simulink model "ofdm_txrx_supermimo"  (Simulink 7.1)
settings: Solver=VariableStepDiscrete  SolverMode=SingleTasking  StartTime=0.0  StopTime=simOnly_simLength  RelTol=1e-3
PostLoadFcn:
  | ofdm_rx_supermimo_init
InitFcn:
  | ofdm_rx_supermimo_init
StartFcn:
  | ofdm_rx_supermimo_init
values from neighbouring files (symbols this model uses):
  Action0_Config = 0* 31*2^18 + 0*2^24 + PHY_AUTORESPONSE_REQ_GOODHDR + PHY_AUTORESPONSE_REQ_GOODPKT + PHY_AUTORESPONSE_REQ_FLAGA    [ofdm_tx_supermimo_init.m]
  Action1_Config = 0* 62*2^18 + PHY_AUTORESPONSE_REQ_GOODHDR + PHY_AUTORESPONSE_REQ_GOODPKT    [ofdm_tx_supermimo_init.m]
  Action2_Config = 0    [ofdm_tx_supermimo_init.m]
  Action3_Config = 0    [ofdm_tx_supermimo_init.m]
  Action4_Config = 0    [ofdm_tx_supermimo_init.m]
  Action5_Config = 0    [ofdm_tx_supermimo_init.m]
  ActionID_AFTransmit = 31    [ofdm_tx_supermimo_init.m]
  ActionID_Disabled = 0    [ofdm_tx_supermimo_init.m]
  ActionID_SetFlagA = 62    [ofdm_tx_supermimo_init.m]
  ActionID_SetFlagB = 61    [ofdm_tx_supermimo_init.m]
  BER_RAM_init_size = 2048    [ofdm_tx_supermimo_init.m]
  CPLength = 16    [ofdm_tx_supermimo_init.m]
  longCorr_coef_i = [-4*real(LTS) 0]    [ofdm_rx_supermimo_init.m]
  longCorr_coef_q = [4*imag(LTS) 0]    [ofdm_rx_supermimo_init.m]
  Match0_Config = 0    [ofdm_tx_supermimo_init.m]
  Match1_Config = 0    [ofdm_tx_supermimo_init.m]
  Match2_Config = 0    [ofdm_tx_supermimo_init.m]
  Match3_Config = 0    [ofdm_tx_supermimo_init.m]
  Match4_Config = 0    [ofdm_tx_supermimo_init.m]
  Match5_Config = 0    [ofdm_tx_supermimo_init.m]
  Match6_Config = 0    [ofdm_tx_supermimo_init.m]
  Match7_Config = 0    [ofdm_tx_supermimo_init.m]
  max_CP_length = 16    [ofdm_tx_supermimo_init.m]
  max_num_baseRateSymbols = 31    [ofdm_tx_supermimo_init.m]
  max_num_subcarriers = 64    [ofdm_tx_supermimo_init.m]
  max_num_trainingSymbols = 15    [ofdm_tx_supermimo_init.m]
  max_numBytes = 16384    [ofdm_tx_supermimo_init.m]
  max_OFDM_symbols = 511    [ofdm_tx_supermimo_init.m]
  modConstellation_qam16 = .75*[1 3 -1 -3]./3    [ofdm_tx_supermimo_init.m]
  modConstellation_qam64 = 0.875*[3 1 5 7 -3 -1 -5 -7]./7    [ofdm_tx_supermimo_init.m]
  modConstellation_qpsk = [1 -1]./sqrt(2)    [ofdm_tx_supermimo_init.m]
  numBaseRateSymbols = ceil(numHeaderBytes / numBytes_BaseRateOFDMSymbol)    [ofdm_tx_supermimo_init.m]
  numSubcarriers = 64    [ofdm_tx_supermimo_init.m]
  numTrainingSymbols = 2    [ofdm_tx_supermimo_init.m]
  pilot_indicies = 7 + ( (64-21) * 2^8) + ( (64-7) * 2^16) + (21 * 2^24)    (= 356068103)    [ofdm_tx_supermimo_init.m]
  pilotValues = hex2dec('A57D') + (2^16 * hex2dec('5A82'))    [ofdm_tx_supermimo_init.m]
  pkt_crossCorr_thresh = 0.7    [ofdm_rx_supermimo_init.m]
  pkt_energy_thresh = 0    [ofdm_rx_supermimo_init.m]
  pktByteNums = numHeaderBytes +  				byteIndex_numPayloadBytes(1)*2^8 +  				byteIndex_numPayloadBytes(2)*2^16 +  				byteIndex_simpleDynModMasks * 2^24    [ofdm_rx_supermimo_init.m]
  PktDet_Delay = 58+32 +      Symbol_Timing_Offset*2^7 +      0*2^16 +      (117* 2^24)    (= 1962935642)    [ofdm_rx_supermimo_init.m]
  pktTiming_count_payloadStart = pktTiming_count_lsStart + pktTiming_ls_num*pktTiming_ls_length    [ofdm_rx_supermimo_init.m]
  preamble = preamble_scale*[shortsyms_10 longsyms_2]    [ofdm_tx_supermimo_init.m]
  preamble_ROM = preamble_I_ROM + preamble_Q_ROM*2^16    [ofdm_tx_supermimo_init.m]
  RAM_init_size = max_numBytes    (= 16384)    [ofdm_tx_supermimo_init.m]
  reg_AF_Tx_Blanking = (320+80) + ( (320+80+79)*2^16)    (= 31392144)    [ofdm_rx_supermimo_init.m]
  reg_AF_Tx_Scaling = 2944    [ofdm_rx_supermimo_init.m]
  reg_Interrupt_PktBuf_Control = reg_InterruptControl +  	1 * 2^16 +  	0 * 2^24    (= 65656)    [ofdm_rx_supermimo_init.m]
  reg_PilotCalcParams = (4 * 2^16) + (minPilotChanMag)    (= 262184.96)    [ofdm_rx_supermimo_init.m]
  regRx_chanEst_minMag = ((0.5)*2^15) + ((0.5 * 2^15)*2^16)    (= 1073758208)    [ofdm_rx_supermimo_init.m]
  regRx_coarseCalcCorrection = round((1.3e-3 - 1.2982e-3)*2^32)    [ofdm_rx_supermimo_init.m]
  regRx_pilotCalcCorrection = round(1.00115 * 2^31)    [ofdm_rx_supermimo_init.m]
  regRx_preCFOoptions = 0 * 2^0 +  	1 * 2^1 +  	0    (= 2)    [ofdm_rx_supermimo_init.m]
  rx_controlBits = 1 * 2  		 + 1 * 4  		 + 0 * 8  		 + 1 * 16 * rx_SISO_Mode  		 + 1 * 32  		 + 1 * 64  		 + 1 * 128  		 + 1 * 256  		 + 1 * 512  		 + 1 * 1024  		 + 1 * 4096  		 + 0 * 16384  		 + 0 * 32768  		 + 1 * 65536  		 + 0 * 131072  		 + 0 * 262144  		 + 1 * 524288  		 + 1 * 1048576 * tx_Alamouti_Mode  		 + 0 * 2097152           + 1 * 33554432  		 + 0 * 4194304  		 + 0 * 8388608  		 + 0 * 16777216  		 + 1 *  ...(+49 chars)    [ofdm_rx_supermimo_init.m]
  Rx_PktDet_LongCorr_Params = LongCorrCounterLoad + 0*2^8 + 0*2^16 + 255*2^24    (= 4278190325)    [ofdm_rx_supermimo_init.m]
  Rx_PktDet_LongCorrThresholds = 8000    [ofdm_rx_supermimo_init.m]
  rx_postEq_scaling = round(rxScaling*2^11) * (1 + 2^16)    [ofdm_rx_supermimo_init.m]
  RxDataScrambling_Seq = [40 198 78 63 82 173 102 245 48 111 172 115 147 230 216 93 72 65 62 2 205 242 122 90 128 83 105 97 73 10 5 252]    [ofdm_tx_supermimo_init.m]
  RxReg_FixedPktLen = 0    [ofdm_rx_supermimo_init.m]
  shortSymbol_time = shortSymbol_time(1:16).'    [ofdm_tx_supermimo_init.m]
  simOnly_numSamples = length(preamble)+( (numSubcarriers+CPLength)*(numTrainingSymbols + numBaseRateSymbols + numFullRateSymbols/2) )    [ofdm_tx_supermimo_init.m]
  train = [train train]    [ofdm_tx_supermimo_init.m]
  tx_controlBits = (antB_preambleShift * 2^4) + (2^1 * tx_Alamouti_Mode) +  	(2^0 * tx_SISO_Mode) + 4*0 + 8*1 + 0*256 + 512*0 + 1024*0 +  	0*hex2dec('1000') + 0*hex2dec('2000') + 0*hex2dec('4000') +  	1*hex2dec('8000') + 1*hex2dec('10000') + hex2dec('20000') +  	16*hex2dec('100000')    [ofdm_tx_supermimo_init.m]
  tx_delays = (50*2^0) + (0*2^8) + (30*2^12)    (= 122930)    [ofdm_tx_supermimo_init.m]
  tx_scaling = (3072) + (12288 * 2^16)    (= 805309440)    [ofdm_tx_supermimo_init.m]
  TxDataScrambling_Seq = [40 198 78 63 82 173 102 245 48 111 172 115 147 230 216 93 72 65 62 2 205 242 122 90 128 83 105 97 73 10 5 252]    [ofdm_tx_supermimo_init.m]
  txfilt_firpm = 0.95*txfilt_firpm./(max(txfilt_firpm))    [ofdm_tx_supermimo_init.m]
  txfilt_intfilt = [0 intfilt(4,4,.8)]    [ofdm_tx_supermimo_init.m]
  txReg_symbolCounts = (2^8 *numBaseRateSymbols ) + numTrainingSymbols    [ofdm_tx_supermimo_init.m]
  TxRx_FFTScaling = (3 * 2^0) +  	(2 * 2^2) +  	(1 * 2^4) +  	(1 * 2^6) +  	(1 * 2^8) +  	(0 * 2^10)    (= 347)    [ofdm_tx_supermimo_init.m]
undefined symbols (not defined in the file or in its folder): AntA, AntB, BaseRate, Down, gcbh, numBuffs, numBytes, Up, xlBlockLoadCallback, xlGetSimulinkPeriod, xlipgetpartsetting
libraries: xbsIndex_r4 x6035, simulink x4, dspsigops x4, commchan3 x2, dspsrcs4 x2, commrflib2 x2
(part 16 of 61)

== system ofdm_txrx_supermimo/OFDM Tx MIMO/OutputBuffers/RAMs ==
  A We : Inport  OutDataType=sfix(16)  OutScaling=2^0
  B We : Inport  Port=2  OutDataType=sfix(16)  OutScaling=2^0
  A OE# : Inport  Port=3  OutDataType=sfix(16)  OutScaling=2^0
  B OE# : Inport  Port=4  OutDataType=sfix(16)  OutScaling=2^0
  Wr Addr : Inport  Port=5  OutDataType=sfix(16)  OutScaling=2^0
  Rd Addr : Inport  Port=6  OutDataType=sfix(16)  OutScaling=2^0
  Rd Vin : Inport  Port=7  OutDataType=sfix(16)  OutScaling=2^0
  Slot : Inport  Port=8  OutDataType=sfix(16)  OutScaling=2^0
  I : Inport  Port=9  OutDataType=sfix(16)  OutScaling=2^0
  Q : Inport  Port=10  OutDataType=sfix(16)  OutScaling=2^0
  Alamouti Encoding : Subsystem
  Delay : xbsIndex_r4/Delay  en=off  latency=1  dbl_ovrd=off  reg_retiming=off  block_type=delay
      settings: infoedit=Hardware notes: A delay line is a chain, each link of which is an SRL16 followed by a flip-flop. If register retiming is enabled, the delay line is a chain of flip-flops.  block_version=8.2.02
  Down Sample{,1..4} (5 alike) : xbsIndex_r4/Down Sample  sample_ratio=2  sample_phase="Last Value of Frame  (most efficient)"  en=off  latency=1  dbl_ovrd=off  block_type=dsamp
      settings: block_version=VER_STRING_GOES_HERE
  Even Syms RAM : Subsystem
  Logical : xbsIndex_r4/Logical  logical_function=AND  inputs=2  en=off  latency=0  precision=Full  arith_type=Unsigned  n_bits=16  bin_pt=0  align_bp=on  dbl_ovrd=off  block_type=logical
      settings: block_version=VER_STRING_GOES_HERE
  Odd Syms RAM : Subsystem
  AntA_I : Outport  OutDataType=sfix(16)  OutScaling=2^0
  AntA_Q : Outport  Port=2  OutDataType=sfix(16)  OutScaling=2^0
  AntB_I : Outport  Port=3  OutDataType=sfix(16)  OutScaling=2^0
  AntB_Q : Outport  Port=4  OutDataType=sfix(16)  OutScaling=2^0
  Rd Vout : Outport  Port=5  OutDataType=sfix(16)  OutScaling=2^0
  wires:
  Odd Syms RAM/2(" Q") -> Down Sample/1
  Odd Syms RAM/1(" I") -> Down Sample1/1
  Rd Addr/1 -> Even Syms RAM/5(Rd Addr), Odd Syms RAM/5(Rd Addr)
  Even Syms RAM/2(" Q") -> Down Sample2/1
  Even Syms RAM/1(" I") -> Down Sample3/1
  Wr Addr/1 -> Even Syms RAM/4(Wr Addr), Odd Syms RAM/4(Wr Addr)
  I/1 -> Even Syms RAM/1(I), Odd Syms RAM/1(I)
  Q/1 -> Even Syms RAM/2(Q), Odd Syms RAM/2(Q)
  A We/1 -> Even Syms RAM/3(We)
  B We/1 -> Odd Syms RAM/3(We)
  B OE#/1 -> Odd Syms RAM/6(OE#)
  A OE#/1 -> Even Syms RAM/6(OE#)
  Down Sample/1 -> Alamouti Encoding/5(OddSyms_Q)
  Down Sample1/1 -> Alamouti Encoding/4(OddSyms_I)
  Down Sample2/1 -> Alamouti Encoding/3(EvenSyms_Q)
  Down Sample3/1 -> Alamouti Encoding/2(EvenSyms_I)
  Rd Vin/1 -> Rd Vout/1
  Alamouti Encoding/1(AntA_I) -> AntA_I/1
  Alamouti Encoding/2(AntA_Q) -> AntA_Q/1
  Alamouti Encoding/3(AntB_I) -> AntB_I/1
  Alamouti Encoding/4(AntB_Q) -> AntB_Q/1
  Slot/1 -> Delay/1
  [TxReg_Alamouti_Mode] -> Down Sample4/1
  Delay/1 -> Logical/2
  Logical/1 -> Alamouti Encoding/1(Slot)
  Down Sample4/1 -> Logical/1

== system ofdm_txrx_supermimo/OFDM Tx MIMO/OutputBuffers/negedge ==
  D : Inport  OutDataType=sfix(16)  OutScaling=2^0
  Delay : xbsIndex_r4/Delay  en=off  latency=1  dbl_ovrd=off  reg_retiming=on  block_type=delay
      settings: infoedit=Hardware notes: A delay line is a chain, each link of which is an SRL16 followed by a flip-flop. If register retiming is enabled, the delay line is a chain of flip-flops.  block_version=VER_STRING_GOES_HERE
  Inverter1 : xbsIndex_r4/Inverter  en=off  latency=0  dbl_ovrd=off  block_type=inv
      settings: block_version=VER_STRING_GOES_HERE
  Logical3 : xbsIndex_r4/Logical  logical_function=AND  inputs=2  en=off  latency=0  precision=Full  arith_type=Unsigned  n_bits=16  bin_pt=0  align_bp=on  dbl_ovrd=off  block_type=logical
      settings: block_version=VER_STRING_GOES_HERE
  Q : Outport  OutDataType=sfix(16)  OutScaling=2^0
  wires:
  Logical3/1 -> Q/1
  Delay/1 -> Logical3/2
  D/1 -> Delay/1, Inverter1/1
  Inverter1/1 -> Logical3/1

== system ofdm_txrx_supermimo/OFDM Tx MIMO/OutputMuxes/Antenna Swapping ==
  Rst : Inport
  PreamIndex : Inport  Port=2
  AntA_I : Inport  Port=3
  AntA_Q : Inport  Port=4
  AntB_I : Inport  Port=5
  AntB_Q : Inport  Port=6
  1LSB : xbsIndex_r4/Slice  Orientation=down  nbits=1  boolean_output=off  mode=Upper Bit Location + Width  bit1=0  base1=LSB of Input  bit0=0  base0=LSB of Input  dbl_ovrd=off  block_type=slice
      settings: infoedit="Extracts a given range of bits from each input sample and presents it at the output.  The output type is ordinarily unsigned with binary point at zero, but can be Boolean when the slice is one bit wide.<P><P>Hardware notes: In hardware this block costs nothing."  block_version=10.1.3
  Concat : xbsIndex_r4/Concat  num_inputs=2  dbl_ovrd=off  block_type=concat
      settings: block_version=10.1.3
  Constant : xbsIndex_r4/Constant  arith_type=Unsigned  const=9*length(shortSymbol_time)  n_bits=8  bin_pt=0  explicit_period=off  period=1  equ=P=C  opselect=C  inp2=PCIN>>17  opr=+  inp1=P  carry=CIN  dbl_ovrd=off  block_type=constant
  Constant1 : xbsIndex_r4/Constant  arith_type=Unsigned  const=ActionID_AFTransmit  n_bits=ceil(log2(ActionID_AFTransmit))  bin_pt=0  explicit_period=off  period=1  equ=P=C  opselect=C  inp2=PCIN>>17  opr=+  inp1=P  carry=CIN  dbl_ovrd=off  block_type=constant
  Convert{,1..3} (4 alike) : xbsIndex_r4/Convert  arith_type=Boolean  n_bits=16  bin_pt=14  quantization=Truncate  overflow=Wrap  latency=0  dbl_ovrd=off  pipeline=off  block_type=convert
      settings: block_version=10.1.3
  Down Sample : xbsIndex_r4/Down Sample  sample_ratio=2  sample_phase="Last Value of Frame  (most efficient)"  en=off  latency=1  dbl_ovrd=off  block_type=dsamp
      settings: block_version=10.1.3
  Down Sample1 : xbsIndex_r4/Down Sample  sample_ratio=2  sample_phase="Last Value of Frame  (most efficient)"  en=off  latency=1  dbl_ovrd=off  block_type=dsamp
      settings: block_version=10.1.3
  Inverter : xbsIndex_r4/Inverter  en=off  latency=0  dbl_ovrd=off  block_type=inv
      settings: xl_area=[0, 0, 0, 0, 0, 0, 0]  block_version=VER_STRING_GOES_HERE
  Logical1 : xbsIndex_r4/Logical  logical_function=AND  inputs=2  en=off  latency=0  precision=Full  arith_type=Unsigned  n_bits=8  bin_pt=2  align_bp=on  dbl_ovrd=off  block_type=logical
      settings: xl_area=[1 0 0 1 0 0 0]  block_version=VER_STRING_GOES_HERE
  Logical2 : xbsIndex_r4/Logical  logical_function=OR  inputs=2  en=off  latency=0  precision=Full  arith_type=Unsigned  n_bits=8  bin_pt=2  align_bp=on  dbl_ovrd=off  block_type=logical
      settings: xl_area=[1 0 0 1 0 0 0]  block_version=VER_STRING_GOES_HERE
  Logical3 : xbsIndex_r4/Logical  logical_function=AND  inputs=2  en=off  latency=0  precision=Full  arith_type=Unsigned  n_bits=8  bin_pt=2  align_bp=on  dbl_ovrd=off  block_type=logical
      settings: xl_area=[1 0 0 1 0 0 0]  block_version=VER_STRING_GOES_HERE
  Mux1 : xbsIndex_r4/Mux  inputs=3  en=off  latency=0  precision=User Defined  arith_type="Signed  (2's comp)"  n_bits=14  bin_pt=13  quantization=Truncate  overflow=Wrap  dbl_ovrd=off  block_type=mux
      settings: xl_area=[7 0 0 14 0 0 0]  block_version=VER_STRING_GOES_HERE
  Mux{2..3,5..6} (4 alike) : xbsIndex_r4/Mux  inputs=2  en=off  latency=0  precision=User Defined  arith_type="Signed  (2's comp)"  n_bits=14  bin_pt=13  quantization=Truncate  overflow=Wrap  dbl_ovrd=off  block_type=mux
      settings: xl_area=[7 0 0 14 0 0 0]  block_version=VER_STRING_GOES_HERE
  Mux4 : xbsIndex_r4/Mux  inputs=3  en=off  latency=0  precision=User Defined  arith_type="Signed  (2's comp)"  n_bits=14  bin_pt=13  quantization=Truncate  overflow=Wrap  dbl_ovrd=off  block_type=mux
      settings: xl_area=[7 0 0 14 0 0 0]  block_version=VER_STRING_GOES_HERE
  PreambleEn : Subsystem
  Relational : xbsIndex_r4/Relational  mode=a>b  en=off  latency=0  dbl_ovrd=off  block_type=relational
      settings: block_version=10.1.3
  Relational1 : xbsIndex_r4/Relational  mode=a=b  en=off  latency=0  dbl_ovrd=off  block_type=relational
      settings: block_version=10.1.3
  Tx : Scope  NumInputPorts=7
      settings: TimeRange=9328  YMin=-0.2~-0.25~-1~-1~0~0~0  YMax=0.15~0.15~1~1~1~3500~1  SaveName=ScopeData7
  done{1..6} (6 alike) : xbsIndex_r4/Gateway Out  hdl_port=off  timing_constraint=None  locs_specified=off  LOCs={}  block_type=gatewayout
      settings: infoedit="Gateway out block.  Converts Xilinx fixed point inputs into ouputs of type Simulink integer, double, or fixed point.<P><P>Hardware notes:  In hardware these blocks become top level output ports or are discarded, depending on how they are configured."  xl_area=[0 0 0 0 1 0 0]  block_version=VER_STRING_GOES_HERE
  posedge3 : Subsystem
  AF Payload : Outport
  " AntA_I" : Outport  Port=2
  " AntA_Q" : Outport  Port=3
  " AntB_I" : Outport  Port=4
  " AntB_Q" : Outport  Port=5
  wires:
  AntA_I/1 -> Mux4/2, Mux3/3, Mux5/2, done4/1
  AntB_I/1 -> Mux4/3, Mux3/2
  PreamIndex/1 -> Relational/1
  Concat/1 -> Down Sample/1
  Mux5/1 -> Mux4/4
  [AF_OutputSamples_I] -> Mux5/3, done3/1
  PreambleEn/1(Q) -> Mux5/1, Mux2/1, Convert3/1
  Mux4/1 -> " AntA_I"/1, done1/1
  Mux1/1 -> " AntA_Q"/1, done2/1
  AntA_Q/1 -> Mux2/2, Mux1/2, Mux6/3, done5/1
  AntB_Q/1 -> Mux1/3, Mux6/2
  Mux2/1 -> Mux1/4
  [AF_OutputSamples_Q] -> Mux2/3, done6/1
  Mux3/1 -> " AntB_I"/1
  Mux6/1 -> " AntB_Q"/1
  1LSB/1 -> Mux3/1, Mux6/1
  Down Sample/1 -> Mux4/1, Mux1/1, 1LSB/1
  [RxAutoReply_Action_BufNum] -> Relational1/1
  Constant1/1 -> Relational1/2
  Relational1/1 -> Inverter/1, Concat/1, Down Sample1/1
  Inverter/1 -> Logical1/1
  Logical1/1 -> Concat/2
  [TxReg_SwapAntennas] -> Convert/1
  Convert/1 -> Logical2/1
  Relational/1 -> posedge3/1(D)
  posedge3/1(Q) -> PreambleEn/2(S)
  Constant/1 -> Relational/2
  Rst/1 -> PreambleEn/1(R)
  Logical3/1 -> AF Payload/1
  [RxAutoReplyProc_SwapAnt] -> Convert1/1
  Convert1/1 -> Logical2/2
  Logical2/1 -> Logical1/2
  done4/1 -> Tx/1
  done5/1 -> Tx/2
  done3/1 -> Tx/3
  done6/1 -> Tx/4
  done1/1 -> Tx/5
  done2/1 -> Tx/6
  Convert2/1 -> Logical3/1
  Convert3/1 -> Logical3/2
  Down Sample1/1 -> Convert2/1

== system ofdm_txrx_supermimo/OFDM Tx MIMO/OutputMuxes/Posedge2 ==
  D : Inport  OutDataType=sfix(16)  OutScaling=2^0
  Delay : xbsIndex_r4/Delay  en=off  latency=1  dbl_ovrd=off  reg_retiming=off  block_type=delay
      settings: infoedit=Hardware notes: A delay line is a chain, each link of which is an SRL16 followed by a flip-flop. If register retiming is enabled, the delay line is a chain of flip-flops.  xl_area=[1 1 0 0 0 0 0]  block_version=VER_STRING_GOES_HERE
  Inverter : xbsIndex_r4/Inverter  en=off  latency=0  dbl_ovrd=off  block_type=inv
      settings: xl_area=[0 0 0 0 0 0 0]  block_version=VER_STRING_GOES_HERE
  Logical : xbsIndex_r4/Logical  logical_function=AND  inputs=2  en=off  latency=0  precision=Full  arith_type=Unsigned  n_bits=1  bin_pt=0  align_bp=on  dbl_ovrd=off  block_type=logical
      settings: xl_area=[1 0 0 1 0 0 0]  block_version=VER_STRING_GOES_HERE
  Q : Outport  OutDataType=sfix(16)  OutScaling=2^0
  wires:
  Delay/1 -> Inverter/1
  Inverter/1 -> Logical/2
  D/1 -> Logical/1, Delay/1
  Logical/1 -> Q/1

== system ofdm_txrx_supermimo/OFDM Tx MIMO/OutputMuxes/Preamble Generation ==
  SampInd : Inport  OutDataType=sfix(16)  OutScaling=2^0
  14LSB : xbsIndex_r4/Slice  nbits=14  boolean_output=off  mode=Lower Bit Location + Width  bit1=0  base1=MSB of Input  bit0=0  base0=LSB of Input  dbl_ovrd=off  block_type=slice
      settings: infoedit="Extracts a given range of bits from each input sample and presents it at the output.  The output type is ordinarily unsigned with binary point at zero, but can be Boolean when the slice is one bit wide.<P><P>Hardware notes: In hardware this block costs nothing."  block_version=10.1.3
  "14LSB " : xbsIndex_r4/Slice  nbits=14  boolean_output=off  mode=Lower Bit Location + Width  bit1=0  base1=MSB of Input  bit0=0  base0=LSB of Input  dbl_ovrd=off  block_type=slice
      settings: infoedit="Extracts a given range of bits from each input sample and presents it at the output.  The output type is ordinarily unsigned with binary point at zero, but can be Boolean when the slice is one bit wide.<P><P>Hardware notes: In hardware this block costs nothing."  block_version=10.1.3
  14LSB+16 : xbsIndex_r4/Slice  nbits=14  boolean_output=off  mode=Lower Bit Location + Width  bit1=0  base1=MSB of Input  bit0=16  base0=LSB of Input  dbl_ovrd=off  block_type=slice
      settings: infoedit="Extracts a given range of bits from each input sample and presents it at the output.  The output type is ordinarily unsigned with binary point at zero, but can be Boolean when the slice is one bit wide.<P><P>Hardware notes: In hardware this block costs nothing."  block_version=10.1.3
  "14LSB+16 " : xbsIndex_r4/Slice  nbits=14  boolean_output=off  mode=Lower Bit Location + Width  bit1=0  base1=MSB of Input  bit0=16  base0=LSB of Input  dbl_ovrd=off  block_type=slice
      settings: infoedit="Extracts a given range of bits from each input sample and presents it at the output.  The output type is ordinarily unsigned with binary point at zero, but can be Boolean when the slice is one bit wide.<P><P>Hardware notes: In hardware this block costs nothing."  block_version=10.1.3
  Addr Gen : Subsystem
  Constant1 : xbsIndex_r4/Constant  arith_type=Unsigned  const=0  n_bits=32  bin_pt=0  explicit_period=off  period=1  equ=P=C  opselect=C  inp2=PCIN>>17  opr=+  inp1=P  carry=CIN  dbl_ovrd=off  block_type=constant
      settings: block_version=8.2.02
  Constant2 : xbsIndex_r4/Constant  arith_type=Boolean  const=0  n_bits=14  bin_pt=13  explicit_period=off  period=1  equ=P=C  opselect=C  inp2=PCIN>>17  opr=+  inp1=P  carry=CIN  dbl_ovrd=off  block_type=constant
      settings: block_version=8.2.02
  Preamble : xbsIndex_r4/Dual Port RAM  depth=length(preamble_ROM)  initVector=preamble_ROM  distributed_mem=Block RAM  init_a=0  init_b=0  rst_a=off  rst_b=on  en_a=off  en_b=off  latency=1  write_mode_A=Read After Write  write_mode_B=Read After Write  dbl_ovrd=off  optimize=Area  use_rpm=on  block_type=dpram
      settings: block_version=8.2.01
  Reinterpret{,1..3} (4 alike) : xbsIndex_r4/Reinterpret  force_arith_type=on  arith_type="Signed  (2's comp)"  force_bin_pt=on  bin_pt=13  block_type=reinterpret
      settings: infoedit="Changes signal type without altering the binary representation.   You can changed the signal between signed and unsigned, and relocate the binary point.<P><P>Hardware notes: In hardware this block costs nothing.<P><P>Example:  Suppose the input is 6 bits wide, signed, with 2 fractional bits, and the output is forced to unsigned with 0 fractional bits.  Then an input of -2.0 (1110.00 in binary 2's complement) becomes an output of 56 (111000 in binary)."  block_version=10.1.3
  AntA_I : Outport  OutDataType=sfix(16)  OutScaling=2^0
  AntA_Q : Outport  Port=2  OutDataType=sfix(16)  OutScaling=2^0
  AntB_I : Outport  Port=3  OutDataType=sfix(16)  OutScaling=2^0
  AntB_Q : Outport  Port=4  OutDataType=sfix(16)  OutScaling=2^0
  wires:
  Constant1/1 -> Preamble/2, Preamble/5
  Constant2/1 -> Preamble/3, Preamble/6
  SampInd/1 -> Addr Gen/1(Samp Ind)
  Preamble/1 -> 14LSB/1, 14LSB+16/1
  14LSB/1 -> Reinterpret/1
  14LSB+16/1 -> Reinterpret1/1
  "14LSB "/1 -> Reinterpret2/1
  "14LSB+16 "/1 -> Reinterpret3/1
  Preamble/2 -> "14LSB "/1, "14LSB+16 "/1
  Reinterpret/1 -> AntA_I/1
  Reinterpret1/1 -> AntA_Q/1
  Reinterpret2/1 -> AntB_I/1
  Addr Gen/2(AddrB) -> Preamble/4
  Addr Gen/1(AddrA) -> Preamble/1
  [TxReg_DisableAntBPreamble] -> Preamble/7
  Reinterpret3/1 -> AntB_Q/1

== system ofdm_txrx_supermimo/OFDM Tx MIMO/OutputMuxes/TxDone Logic ==
  note: Delay to allow final samples to leave pipeline
      | before Tx gets disabled
  Pkt Done : Inport  OutDataType=sfix(16)  OutScaling=2^0
  Copy_Downsample : Subsystem
  Delay4 : xbsIndex_r4/Delay  en=off  latency=10  dbl_ovrd=off  reg_retiming=off  block_type=delay
      settings: infoedit=Hardware notes: A delay line is a chain, each link of which is an SRL16 followed by a flip-flop. If register retiming is enabled, the delay line is a chain of flip-flops.  block_version=VER_STRING_GOES_HERE
  Logical3 : xbsIndex_r4/Logical  logical_function=OR  inputs=2  en=off  latency=0  precision=Full  arith_type=Unsigned  n_bits=8  bin_pt=2  align_bp=on  dbl_ovrd=off  block_type=logical
      settings: xl_area=[1 0 0 1 0 0 0]  block_version=VER_STRING_GOES_HERE
  Logical4 : xbsIndex_r4/Logical  logical_function=OR  inputs=2  en=off  latency=0  precision=Full  arith_type=Unsigned  n_bits=8  bin_pt=2  align_bp=on  dbl_ovrd=off  block_type=logical
      settings: xl_area=[1 0 0 1 0 0 0]  block_version=VER_STRING_GOES_HERE
  Done : Outport
  wires:
  [TxReset] -> Logical3/1
  Pkt Done/1 -> Logical3/2
  [AF_TxDone] -> Logical4/1
  Logical3/1 -> Copy_Downsample/1(D)
  Copy_Downsample/1(Q) -> Logical4/2
  Logical4/1 -> Delay4/1
  Delay4/1 -> Done/1

== system ofdm_txrx_supermimo/OFDM Tx MIMO/OutputMuxes/TxRunning Outputs ==
  Tx : Inport
  Convert : xbsIndex_r4/Convert  arith_type=Unsigned  n_bits=1  bin_pt=0  quantization=Truncate  overflow=Wrap  latency=0  dbl_ovrd=off  pipeline=off  block_type=convert
      settings: xl_area=[0, 0, 0, 0, 0, 0, 0]  block_version=VER_STRING_GOES_HERE
  Convert1 : xbsIndex_r4/Convert  arith_type=Unsigned  n_bits=1  bin_pt=0  quantization=Truncate  overflow=Wrap  latency=0  dbl_ovrd=off  pipeline=off  block_type=convert
      settings: xl_area=[0, 0, 0, 0, 0, 0, 0]  block_version=VER_STRING_GOES_HERE
  Convert3 : xbsIndex_r4/Convert  arith_type=Unsigned  n_bits=1  bin_pt=0  quantization=Truncate  overflow=Wrap  latency=0  dbl_ovrd=off  pipeline=off  block_type=convert
      settings: xl_area=[0, 0, 0, 0, 0, 0, 0]  block_version=VER_STRING_GOES_HERE
  Delay : Subsystem
  Down Sample6 : xbsIndex_r4/Down Sample  sample_ratio=2  sample_phase="Last Value of Frame  (most efficient)"  en=off  latency=1  dbl_ovrd=off  block_type=dsamp
      settings: block_version=VER_STRING_GOES_HERE
  Logical1 : xbsIndex_r4/Logical  logical_function=AND  inputs=2  en=off  latency=0  precision=Full  arith_type=Unsigned  n_bits=16  bin_pt=0  align_bp=on  dbl_ovrd=off  block_type=logical
  Logical2 : xbsIndex_r4/Logical  logical_function=AND  inputs=2  en=off  latency=0  precision=Full  arith_type=Unsigned  n_bits=16  bin_pt=0  align_bp=on  dbl_ovrd=off  block_type=logical
  Payload Running : xbsIndex_r4/Register  init=0  rst=off  en=off  dbl_ovrd=off  block_type=register
      settings: xl_area=[1 1 0 0 0 0 0]  block_version=VER_STRING_GOES_HERE
  Payload Running1 : xbsIndex_r4/Register  init=0  rst=off  en=off  dbl_ovrd=off  block_type=register
      settings: xl_area=[1 1 0 0 0 0 0]  block_version=VER_STRING_GOES_HERE
  TxRunning : Scope  NumInputPorts=7
      settings: TimeRange=9328  YMin=-0.2~-0.25~-1~-1~0~0~0  YMax=0.15~0.15~1~1~1~3500~1  SaveName=ScopeData5
  Tx_debug_pktRunning : xbsIndex_r4/Gateway Out  hdl_port=on  timing_constraint=None  locs_specified=off  LOCs={}  block_type=gatewayout
      settings: infoedit="Gateway out block.  Converts Xilinx fixed point inputs into ouputs of type Simulink integer, double, or fixed point.<P><P>Hardware notes:  In hardware these blocks become top level output ports or are discarded, depending on how they are configured."  xl_area=[0, 0, 0, 0, 0, 0, 0]  sginterface={'Xilinx'=>{'jtaghwcosim'=>{'non_memory_mapped_port'=>'debug_pktRunning'}}}  block_version=VER_STRING_GOES_HERE
  Tx_pktRunning_d0 : xbsIndex_r4/Gateway Out  hdl_port=on  timing_constraint=None  locs_specified=off  LOCs={}  block_type=gatewayout
      settings: infoedit="Gateway out block.  Converts Xilinx fixed point inputs into ouputs of type Simulink integer, double, or fixed point.<P><P>Hardware notes:  In hardware these blocks become top level output ports or are discarded, depending on how they are configured."  xl_area=[0, 0, 0, 0, 0, 0, 0]  sginterface={'Xilinx'=>{'jtaghwcosim'=>{'non_memory_mapped_port'=>'debug_pktRunning'}}}  block_version=VER_STRING_GOES_HERE
  Tx_pktRunning_d1 : xbsIndex_r4/Gateway Out  hdl_port=on  timing_constraint=None  locs_specified=off  LOCs={}  block_type=gatewayout
      settings: infoedit="Gateway out block.  Converts Xilinx fixed point inputs into ouputs of type Simulink integer, double, or fixed point.<P><P>Hardware notes:  In hardware these blocks become top level output ports or are discarded, depending on how they are configured."  xl_area=[0, 0, 0, 0, 0, 0, 0]  sginterface={'Xilinx'=>{'jtaghwcosim'=>{'non_memory_mapped_port'=>'debug_pktRunning'}}}  block_version=VER_STRING_GOES_HERE
  Up Sample : xbsIndex_r4/Up Sample  sample_ratio=4  copy_samples=on  dbl_ovrd=off  block_type=usamp
      settings: infoedit="Up samples input data.  Inserted values  can be zeros or copies of the most recent input sample.<P><P>Hardware notes: No hardware is needed if inserted values are copies of the input sample; otherwise, a mux and single bit flip-flop are used."  block_version=8.2.02
  Up Sample1 : xbsIndex_r4/Up Sample  sample_ratio=4  copy_samples=on  dbl_ovrd=off  block_type=usamp
      settings: infoedit="Up samples input data.  Inserted values  can be zeros or copies of the most recent input sample.<P><P>Hardware notes: No hardware is needed if inserted values are copies of the input sample; otherwise, a mux and single bit flip-flop are used."  block_version=8.2.02
  done{1..6} (6 alike) : xbsIndex_r4/Gateway Out  hdl_port=off  timing_constraint=None  locs_specified=off  LOCs={}  block_type=gatewayout
      settings: infoedit="Gateway out block.  Converts Xilinx fixed point inputs into ouputs of type Simulink integer, double, or fixed point.<P><P>Hardware notes:  In hardware these blocks become top level output ports or are discarded, depending on how they are configured."  xl_area=[0 0 0 0 1 0 0]  block_version=VER_STRING_GOES_HERE
  posedge2 : Subsystem
  wires:
  Up Sample/1 -> Tx_debug_pktRunning/1, done5/1
  Tx/1 -> posedge2/1(In), Convert/1, done4/1
  posedge2/1(Out) -> Delay/1(Tx)
  [TxReset] -> Down Sample6/1
  Payload Running/1 -> Tx_pktRunning_d1/1, done3/1
  Logical1/1 -> Convert1/1
  Logical2/1 -> Convert3/1
  [TxReg_TxRunningOut_en1] -> Logical1/2
  [TxReg_TxRunningOut_en0] -> Logical2/2
  Payload Running1/1 -> Tx_pktRunning_d0/1, done6/1
  Convert1/1 -> Payload Running/1
  Convert3/1 -> Payload Running1/1
  Convert/1 -> Up Sample/1
  Down Sample6/1 -> Delay/2(Rst)
  Delay/1(Tx_d) -> Up Sample1/1
  Up Sample1/1 -> Logical2/1, Logical1/1, done1/1
  done4/1 -> TxRunning/1
  done5/1 -> TxRunning/2
  Delay/2(dly) -> done2/1
  done1/1 -> TxRunning/3
  done2/1 -> TxRunning/4
  done3/1 -> TxRunning/5
  done6/1 -> TxRunning/6

== system ofdm_txrx_supermimo/OFDM Tx MIMO/Pre-Spin,  Filters & DACs/A*conj(B) ==
  Re[A] : Inport  OutDataType=sfix(16)  OutScaling=2^0
  Im[A] : Inport  Port=2  OutDataType=sfix(16)  OutScaling=2^0
  Im[B] : Inport  Port=3  OutDataType=sfix(16)  OutScaling=2^0
  Re[B] : Inport  Port=4  OutDataType=sfix(16)  OutScaling=2^0
  Add1 : xbsIndex_r4/AddSub  mode=Addition  use_carryin=off  use_carryout=off  en=off  latency=0  precision=User Defined  arith_type="Signed  (2's comp)"  n_bits=16  bin_pt=15  quantization=Truncate  overflow=Wrap  dbl_ovrd=off  use_behavioral_HDL=on  pipelined=off  use_rpm=on  hw_selection=Fabric  block_type=addsub
      settings: xl_area=[9 0 0 16 0 0 0]  block_version=VER_STRING_GOES_HERE
  Counter : xbsIndex_r4/Counter  Orientation=down  cnt_type=Free Running  cnt_to=4  operation=Up  start_count=0  cnt_by_val=1  arith_type=Unsigned  n_bits=2  bin_pt=0  load_pin=off  rst=off  en=off  explicit_period=on  period=1  dbl_ovrd=off  use_behavioral_HDL=off  use_rpm=on  implementation=Fabric  block_type=counter
      settings: xl_area=[3 3 0 4 0 0 0]  block_version=VER_STRING_GOES_HERE
  Down Sample3 : xbsIndex_r4/Down Sample  sample_ratio=4  sample_phase=First Value of Frame  en=off  latency=1  dbl_ovrd=off  block_type=dsamp
      settings: xl_area=[9 17 0 0 0 0 0]  block_version=VER_STRING_GOES_HERE
  Down Sample4 : xbsIndex_r4/Down Sample  sample_ratio=4  sample_phase=First Value of Frame  en=off  latency=1  dbl_ovrd=off  block_type=dsamp
      settings: xl_area=[9 17 0 0 0 0 0]  block_version=VER_STRING_GOES_HERE
  Mult4 : xbsIndex_r4/Mult  precision=User Defined  arith_type="Signed  (2's comp)"  n_bits=17  bin_pt=16  quantization=Truncate  overflow=Wrap  en=off  latency=1  dbl_ovrd=off  use_behavioral_HDL=off  use_embedded=on  opt=Speed  optimum_pipeline=off  pipeline=off  use_rpm=on  placement_style=Rectangular shape  block_type=mult
      settings: infoedit=Hardware notes: To use the internal pipeline stage of the dedicated multiplier you must select 'Pipeline for maximum performance'.  xl_area=[14 27 0 27 0 1 0]  block_version=VER_STRING_GOES_HERE
  Mux : xbsIndex_r4/Mux  inputs=4  en=off  latency=0  precision=Full  arith_type="Signed  (2's comp)"  n_bits=16  bin_pt=13  quantization=Truncate  overflow=Wrap  dbl_ovrd=off  block_type=mux
      settings: xl_area=[14 0 0 28 0 0 0]  block_version=VER_STRING_GOES_HERE
  Mux1 : xbsIndex_r4/Mux  inputs=4  en=off  latency=0  precision=Full  arith_type="Signed  (2's comp)"  n_bits=16  bin_pt=13  quantization=Truncate  overflow=Wrap  dbl_ovrd=off  block_type=mux
      settings: xl_area=[14 0 0 28 0 0 0]  block_version=VER_STRING_GOES_HERE
  Negate : xbsIndex_r4/Negate  precision=Full  arith_type="Signed  (2's comp)"  n_bits=14  bin_pt=13  quantization=Truncate  overflow=Flag as error  en=off  latency=0  dbl_ovrd=off  block_type=negate
      settings: xl_area=[7 0 0 14 0 0 0]  block_version=VER_STRING_GOES_HERE
  Negate1 : xbsIndex_r4/Negate  precision=Full  arith_type="Signed  (2's comp)"  n_bits=14  bin_pt=13  quantization=Truncate  overflow=Flag as error  en=off  latency=0  dbl_ovrd=off  block_type=negate
      settings: xl_area=[7 0 0 14 0 0 0]  block_version=VER_STRING_GOES_HERE
  Register : xbsIndex_r4/Register  init=0  rst=off  en=off  dbl_ovrd=off  block_type=register
      settings: block_version=10.1.2
  Register1 : xbsIndex_r4/Register  init=0  rst=off  en=off  dbl_ovrd=off  block_type=register
      settings: block_version=10.1.2
  Register2 : xbsIndex_r4/Register  init=0  rst=off  en=off  dbl_ovrd=off  block_type=register
      settings: block_version=10.1.2
  Slice : xbsIndex_r4/Slice  nbits=2  boolean_output=off  mode=Lower Bit Location + Width  bit1=0  base1=MSB of Input  bit0=0  base0=LSB of Input  dbl_ovrd=off  block_type=slice
      settings: infoedit="Extracts a given range of bits from each input sample and presents it at the output.  The output type is ordinarily unsigned with binary point at zero, but can be Boolean when the slice is one bit wide.<P><P>Hardware notes: In hardware this block costs nothing."  block_version=VER_STRING_GOES_HERE
  Up Sample{1..4} (4 alike) : xbsIndex_r4/Up Sample  sample_ratio=4  copy_samples=on  dbl_ovrd=off  block_type=usamp
      settings: infoedit="Up samples input data.  Inserted values  can be zeros or copies of the most recent input sample.<P><P>Hardware notes: No hardware is needed if inserted values are copies of the input sample; otherwise, a mux and single bit flip-flop are used."  xl_area=[0 0 0 0 0 0 0]  block_version=VER_STRING_GOES_HERE
  Re : Outport  OutDataType=sfix(16)  OutScaling=2^0
  Im : Outport  Port=2  OutDataType=sfix(16)  OutScaling=2^0
  wires:
  Slice/1 -> Mux/1, Mux1/1
  Counter/1 -> Slice/1
  Register2/1 -> Add1/2
  Mult4/1 -> Add1/1, Register2/1
  Up Sample1/1 -> Mux1/5, Negate/1
  Up Sample4/1 -> Negate1/1
  Up Sample2/1 -> Mux/2, Mux/5
  Up Sample3/1 -> Mux1/4, Mux1/2
  Re[A]/1 -> Up Sample3/1
  Re[B]/1 -> Up Sample2/1
  Im[A]/1 -> Up Sample1/1
  Im[B]/1 -> Up Sample4/1
  Mux1/1 -> Mult4/1
  Mux/1 -> Mult4/2
  Register/1 -> Register1/1
  Down Sample4/1 -> Im/1
  Down Sample3/1 -> Re/1
  Add1/1 -> Register/1, Down Sample4/1
  Negate/1 -> Mux1/3
  Register1/1 -> Down Sample3/1
  Negate1/1 -> Mux/4, Mux/3

== system ofdm_txrx_supermimo/OFDM Tx MIMO/Pre-Spin,  Filters & DACs/A*conj(B)  ==
  Re[B] : Inport  OutDataType=sfix(16)  OutScaling=2^0
  Im[B] : Inport  Port=2  OutDataType=sfix(16)  OutScaling=2^0
  Re[A] : Inport  Port=3  OutDataType=sfix(16)  OutScaling=2^0
  Im[A] : Inport  Port=4  OutDataType=sfix(16)  OutScaling=2^0
  Add1 : xbsIndex_r4/AddSub  mode=Addition  use_carryin=off  use_carryout=off  en=off  latency=0  precision=User Defined  arith_type="Signed  (2's comp)"  n_bits=16  bin_pt=15  quantization=Truncate  overflow=Wrap  dbl_ovrd=off  use_behavioral_HDL=on  pipelined=off  use_rpm=on  hw_selection=Fabric  block_type=addsub
      settings: xl_area=[9 0 0 16 0 0 0]  block_version=VER_STRING_GOES_HERE
  Counter : xbsIndex_r4/Counter  Orientation=down  cnt_type=Free Running  cnt_to=4  operation=Up  start_count=0  cnt_by_val=1  arith_type=Unsigned  n_bits=2  bin_pt=0  load_pin=off  rst=off  en=off  explicit_period=on  period=1  dbl_ovrd=off  use_behavioral_HDL=off  use_rpm=on  implementation=Fabric  block_type=counter
      settings: xl_area=[3 3 0 4 0 0 0]  block_version=VER_STRING_GOES_HERE
  Down Sample3 : xbsIndex_r4/Down Sample  sample_ratio=4  sample_phase=First Value of Frame  en=off  latency=1  dbl_ovrd=off  block_type=dsamp
      settings: xl_area=[9 17 0 0 0 0 0]  block_version=VER_STRING_GOES_HERE
  Down Sample4 : xbsIndex_r4/Down Sample  sample_ratio=4  sample_phase=First Value of Frame  en=off  latency=1  dbl_ovrd=off  block_type=dsamp
      settings: xl_area=[9 17 0 0 0 0 0]  block_version=VER_STRING_GOES_HERE
  Mult4 : xbsIndex_r4/Mult  precision=User Defined  arith_type="Signed  (2's comp)"  n_bits=17  bin_pt=16  quantization=Truncate  overflow=Wrap  en=off  latency=1  dbl_ovrd=off  use_behavioral_HDL=off  use_embedded=on  opt=Speed  optimum_pipeline=off  pipeline=off  use_rpm=on  placement_style=Rectangular shape  block_type=mult
      settings: infoedit=Hardware notes: To use the internal pipeline stage of the dedicated multiplier you must select 'Pipeline for maximum performance'.  xl_area=[14 27 0 27 0 1 0]  block_version=VER_STRING_GOES_HERE
  Mux : xbsIndex_r4/Mux  inputs=4  en=off  latency=0  precision=Full  arith_type="Signed  (2's comp)"  n_bits=16  bin_pt=13  quantization=Truncate  overflow=Wrap  dbl_ovrd=off  block_type=mux
      settings: xl_area=[14 0 0 28 0 0 0]  block_version=VER_STRING_GOES_HERE
  Mux1 : xbsIndex_r4/Mux  inputs=4  en=off  latency=0  precision=Full  arith_type="Signed  (2's comp)"  n_bits=16  bin_pt=13  quantization=Truncate  overflow=Wrap  dbl_ovrd=off  block_type=mux
      settings: xl_area=[14 0 0 28 0 0 0]  block_version=VER_STRING_GOES_HERE
  Negate : xbsIndex_r4/Negate  precision=Full  arith_type="Signed  (2's comp)"  n_bits=14  bin_pt=13  quantization=Truncate  overflow=Flag as error  en=off  latency=0  dbl_ovrd=off  block_type=negate
      settings: xl_area=[7 0 0 14 0 0 0]  block_version=VER_STRING_GOES_HERE
  Negate1 : xbsIndex_r4/Negate  precision=Full  arith_type="Signed  (2's comp)"  n_bits=14  bin_pt=13  quantization=Truncate  overflow=Flag as error  en=off  latency=0  dbl_ovrd=off  block_type=negate
      settings: xl_area=[7 0 0 14 0 0 0]  block_version=VER_STRING_GOES_HERE
  Register : xbsIndex_r4/Register  init=0  rst=off  en=off  dbl_ovrd=off  block_type=register
      settings: block_version=10.1.2
  Register1 : xbsIndex_r4/Register  init=0  rst=off  en=off  dbl_ovrd=off  block_type=register
      settings: block_version=10.1.2
  Register2 : xbsIndex_r4/Register  init=0  rst=off  en=off  dbl_ovrd=off  block_type=register
      settings: block_version=10.1.2
  Slice : xbsIndex_r4/Slice  nbits=2  boolean_output=off  mode=Lower Bit Location + Width  bit1=0  base1=MSB of Input  bit0=0  base0=LSB of Input  dbl_ovrd=off  block_type=slice
      settings: infoedit="Extracts a given range of bits from each input sample and presents it at the output.  The output type is ordinarily unsigned with binary point at zero, but can be Boolean when the slice is one bit wide.<P><P>Hardware notes: In hardware this block costs nothing."  block_version=VER_STRING_GOES_HERE
  Up Sample{1..4} (4 alike) : xbsIndex_r4/Up Sample  sample_ratio=4  copy_samples=on  dbl_ovrd=off  block_type=usamp
      settings: infoedit="Up samples input data.  Inserted values  can be zeros or copies of the most recent input sample.<P><P>Hardware notes: No hardware is needed if inserted values are copies of the input sample; otherwise, a mux and single bit flip-flop are used."  xl_area=[0 0 0 0 0 0 0]  block_version=VER_STRING_GOES_HERE
  Re : Outport  OutDataType=sfix(16)  OutScaling=2^0
  Im : Outport  Port=2  OutDataType=sfix(16)  OutScaling=2^0
  wires:
  Register1/1 -> Down Sample3/1
  Negate/1 -> Mux1/3
  Add1/1 -> Down Sample4/1, Register/1
  Down Sample3/1 -> Re/1
  Down Sample4/1 -> Im/1
  Register/1 -> Register1/1
  Mux/1 -> Mult4/2
  Mux1/1 -> Mult4/1
  Im[B]/1 -> Up Sample4/1
  Im[A]/1 -> Up Sample1/1
  Re[B]/1 -> Up Sample2/1
  Re[A]/1 -> Up Sample3/1
  Up Sample3/1 -> Mux1/2, Mux1/4
  Up Sample2/1 -> Mux/5, Mux/2
  Up Sample4/1 -> Negate1/1
  Up Sample1/1 -> Negate/1, Mux1/5
  Mult4/1 -> Register2/1, Add1/1
  Register2/1 -> Add1/2
  Counter/1 -> Slice/1
  Slice/1 -> Mux1/1, Mux/1
  Negate1/1 -> Mux/3, Mux/4

== system ofdm_txrx_supermimo/OFDM Tx MIMO/Pre-Spin,  Filters & DACs/Filters ==
  I_A : Inport
  Q_A : Inport  Port=2
  I_B : Inport  Port=3
  Q_B : Inport  Port=4
  Interp Filt : Subsystem  mask{h=txfilt_intfilt, polyphaseBranches=4, data_samplePeriod=4, coeff_prec=14, coeff_bp=13, multLatency=1, mult_prec=18, mult_bp=17, add_prec=16, add_bp=15}
      mask prompts: h "Filter Coefficients"; polyphaseBranches "Interpolation Rate"; data_samplePeriod "Input Sample Period"; coeff_prec "Coefficients - Num Bits"; coeff_bp "Coefficients - Binary Point"; multLatency "Multiplier Latency"; mult_prec "Multiplier - Num Bits"; mult_bp "Multiplier - Binary Point"; add_prec "Final Adder - Num Bits"; add_bp "Final Adder - Binary Point"
      mask help:
      | This block implements a interpolating polyphase FIR filter. In this version,
      | the interpolation rate must be 8, and the filter must be of length 64.
      mask init:
      | subFilterLength = 4;
  Interp Filt1 : Subsystem  mask{h=txfilt_firpm, polyphaseBranches=4, data_samplePeriod=4, coeff_prec=14, coeff_bp=13, multLatency=1, mult_prec=18, mult_bp=17, add_prec=16, add_bp=15}
      mask prompts: h "Filter Coefficients"; polyphaseBranches "Interpolation Rate"; data_samplePeriod "Input Sample Period"; coeff_prec "Coefficients - Num Bits"; coeff_bp "Coefficients - Binary Point"; multLatency "Multiplier Latency"; mult_prec "Multiplier - Num Bits"; mult_bp "Multiplier - Binary Point"; add_prec "Final Adder - Num Bits"; add_bp "Final Adder - Binary Point"
      mask help:
      | This block implements a interpolating polyphase FIR filter. In this version,
      | the interpolation rate must be 8, and the filter must be of length 64.
      mask init:
      | subFilterLength = 4;
  Interp Filt2 : Subsystem  mask{h=txfilt_intfilt, polyphaseBranches=4, data_samplePeriod=4, coeff_prec=14, coeff_bp=13, multLatency=1, mult_prec=18, mult_bp=17, add_prec=16, add_bp=15}
      mask prompts: h "Filter Coefficients"; polyphaseBranches "Interpolation Rate"; data_samplePeriod "Input Sample Period"; coeff_prec "Coefficients - Num Bits"; coeff_bp "Coefficients - Binary Point"; multLatency "Multiplier Latency"; mult_prec "Multiplier - Num Bits"; mult_bp "Multiplier - Binary Point"; add_prec "Final Adder - Num Bits"; add_bp "Final Adder - Binary Point"
      mask help:
      | This block implements a interpolating polyphase FIR filter. In this version,
      | the interpolation rate must be 8, and the filter must be of length 64.
      mask init:
      | subFilterLength = 4;
  Interp Filt3 : Subsystem  mask{h=txfilt_intfilt, polyphaseBranches=4, data_samplePeriod=4, coeff_prec=14, coeff_bp=13, multLatency=1, mult_prec=18, mult_bp=17, add_prec=16, add_bp=15}
      mask prompts: h "Filter Coefficients"; polyphaseBranches "Interpolation Rate"; data_samplePeriod "Input Sample Period"; coeff_prec "Coefficients - Num Bits"; coeff_bp "Coefficients - Binary Point"; multLatency "Multiplier Latency"; mult_prec "Multiplier - Num Bits"; mult_bp "Multiplier - Binary Point"; add_prec "Final Adder - Num Bits"; add_bp "Final Adder - Binary Point"
      mask help:
      | This block implements a interpolating polyphase FIR filter. In this version,
      | the interpolation rate must be 8, and the filter must be of length 64.
      mask init:
      | subFilterLength = 4;
  Interp Filt4 : Subsystem  mask{h=txfilt_intfilt, polyphaseBranches=4, data_samplePeriod=4, coeff_prec=14, coeff_bp=13, multLatency=1, mult_prec=18, mult_bp=17, add_prec=16, add_bp=15}
      mask prompts: h "Filter Coefficients"; polyphaseBranches "Interpolation Rate"; data_samplePeriod "Input Sample Period"; coeff_prec "Coefficients - Num Bits"; coeff_bp "Coefficients - Binary Point"; multLatency "Multiplier Latency"; mult_prec "Multiplier - Num Bits"; mult_bp "Multiplier - Binary Point"; add_prec "Final Adder - Num Bits"; add_bp "Final Adder - Binary Point"
      mask help:
      | This block implements a interpolating polyphase FIR filter. In this version,
      | the interpolation rate must be 8, and the filter must be of length 64.
      mask init:
      | subFilterLength = 4;
  Interp Filt5 : Subsystem  mask{h=txfilt_firpm, polyphaseBranches=4, data_samplePeriod=4, coeff_prec=14, coeff_bp=13, multLatency=1, mult_prec=18, mult_bp=17, add_prec=16, add_bp=15}
      mask prompts: h "Filter Coefficients"; polyphaseBranches "Interpolation Rate"; data_samplePeriod "Input Sample Period"; coeff_prec "Coefficients - Num Bits"; coeff_bp "Coefficients - Binary Point"; multLatency "Multiplier Latency"; mult_prec "Multiplier - Num Bits"; mult_bp "Multiplier - Binary Point"; add_prec "Final Adder - Num Bits"; add_bp "Final Adder - Binary Point"
      mask help:
      | This block implements a interpolating polyphase FIR filter. In this version,
      | the interpolation rate must be 8, and the filter must be of length 64.
      mask init:
      | subFilterLength = 4;
  Mux1 : xbsIndex_r4/Mux  inputs=2  en=off  latency=0  precision=Full  arith_type=Unsigned  n_bits=16  bin_pt=14  quantization=Truncate  overflow=Wrap  dbl_ovrd=off  block_type=mux
      settings: block_version=VER_STRING_GOES_HERE
  Mux6 : xbsIndex_r4/Mux  inputs=2  en=off  latency=0  precision=Full  arith_type=Unsigned  n_bits=16  bin_pt=14  quantization=Truncate  overflow=Wrap  dbl_ovrd=off  block_type=mux
      settings: block_version=VER_STRING_GOES_HERE
  " I_A" : Outport
  " Q_A" : Outport  Port=2
  " I_B" : Outport  Port=3
  " Q_B" : Outport  Port=4
  wires:
  Q_A/1 -> Interp Filt/1(X), Interp Filt1/1(X)
  Mux1/1 -> " Q_A"/1
  Interp Filt/1(Y) -> Mux1/2
  Interp Filt1/1(Y) -> Mux1/3
  I_B/1 -> Interp Filt2/1(X)
  Interp Filt2/1(Y) -> " I_B"/1
  Q_B/1 -> Interp Filt3/1(X)
  Interp Filt3/1(Y) -> " Q_B"/1
  I_A/1 -> Interp Filt5/1(X), Interp Filt4/1(X)
  Mux6/1 -> " I_A"/1
  [TxReg_FilterSel] -> Mux6/1, Mux1/1
  Interp Filt4/1(Y) -> Mux6/2
  Interp Filt5/1(Y) -> Mux6/3

== system ofdm_txrx_supermimo/OFDM Tx MIMO/Training_Data/Ant Selector ==
  note: In SISO and multiplexing modes, antenna selction should switch with
      | alternate OFDM symbols during full-rate paylod symbols. During training
      | and base rate symbols there is no need to switch.
      | In Alamouti mode, the selection switches during base-rate
      | and full-rate symbols.
  BaseRate : Inport  OutDataType=sfix(16)  OutScaling=2^0
  CP#_Data : Inport  Port=2  OutDataType=sfix(16)  OutScaling=2^0
  Rst : Inport  Port=3  OutDataType=sfix(16)  OutScaling=2^0
  Inverter2 : xbsIndex_r4/Inverter  en=off  latency=0  dbl_ovrd=off  block_type=inv
      settings: block_version=VER_STRING_GOES_HERE
  Logical1 : xbsIndex_r4/Logical  logical_function=AND  inputs=2  en=off  latency=0  precision=Full  arith_type=Unsigned  n_bits=16  bin_pt=0  align_bp=on  dbl_ovrd=off  block_type=logical
      settings: block_version=VER_STRING_GOES_HERE
  Logical2 : xbsIndex_r4/Logical  logical_function=OR  inputs=2  en=off  latency=0  precision=Full  arith_type=Unsigned  n_bits=16  bin_pt=0  align_bp=on  dbl_ovrd=off  block_type=logical
      settings: block_version=VER_STRING_GOES_HERE
  Oscillator : Subsystem
  negedge : Subsystem
  Sel : Outport  OutDataType=sfix(16)  OutScaling=2^0
  wires:
  CP#_Data/1 -> negedge/1(D)
  Oscillator/1(Q) -> Sel/1
  negedge/1(Q) -> Logical1/2
  Inverter2/1 -> Logical2/2
  Rst/1 -> Oscillator/1(rst)
  BaseRate/1 -> Inverter2/1
  Logical1/1 -> Oscillator/2(en)
  [TxReg_Alamouti_Mode] -> Logical2/1
  Logical2/1 -> Logical1/1
